simulink model "ofdm_txrx_alamouti"  (Simulink 6.3)
settings: Solver=ode45  StartTime=0.0  StopTime=45000  RelTol=1e-3
InitFcn:
  | ofdm_rx_alamouti_init
StartFcn:
  | ofdm_rx_alamouti_init
values from neighbouring files (symbols this model uses):
  CPLength = 16    [ofdm_tx_alamouti_init.m]
  CRCPolynomial = hex2dec('04c11db7')    [calcTxCRC.m]    (also in ofdm_tx_alamouti_init.m)
  hi = zeros(1,length(cci))    [ofdm_rx_alamouti_init.m]
  hr = zeros(1,length(ccr))    [ofdm_rx_alamouti_init.m]
  max_CP_length = 16    [ofdm_tx_alamouti_init.m]
  max_num_baseRateSymbols = 31    [ofdm_tx_alamouti_init.m]
  max_num_subcarriers = 64    [ofdm_tx_alamouti_init.m]
  max_num_trainingSymbols = 15    [ofdm_tx_alamouti_init.m]
  max_OFDM_symbols = 2047    [ofdm_tx_alamouti_init.m]
  modConstellation_qam16 = 0.75*[1 3 -1 -3]./3    [ofdm_tx_alamouti_init.m]
  modConstellation_qam256 = 0.9375*[3 1 5 7 11 9 13 15 -3 -1 -5 -7 -11 -9 -13 -15]./15    [ofdm_tx_alamouti_init.m]
  modConstellation_qam64 = 0.875*[3 1 5 7 -3 -1 -5 -7]./7    [ofdm_tx_alamouti_init.m]
  modConstellation_qpsk = [1 -1]./sqrt(2)    [ofdm_tx_alamouti_init.m]
  numBaseRateSymbols = 2    [ofdm_tx_alamouti_init.m]
  numFullRateSymbols = numFullRateSymbols + mod(numFullRateSymbols, 2)    [ofdm_tx_alamouti_init.m]
  numSubcarriers = 64    [ofdm_tx_alamouti_init.m]
  numTrainingSymbols = 4    [ofdm_tx_alamouti_init.m]
  pkt_crossCorr_thresh = 0.7    [ofdm_rx_alamouti_init.m]
  pkt_energy_thresh = 0    [ofdm_rx_alamouti_init.m]
  pkt_numPayloadBytes = 24+4+20+20+500    (= 568)    [ofdm_tx_alamouti_init.m]
  pktTiming_count_payloadStart = pktTiming_count_lsStart + pktTiming_ls_num*pktTiming_ls_length    [ofdm_rx_init_packetTimingControl.m]
  preamble = 6*[0 shortsyms_10 longsyms_2]    [ofdm_tx_alamouti_init.m]
  preamble_I = real(preamble)    [ofdm_tx_alamouti_init.m]
  preamble_Q = imag(preamble)    [ofdm_tx_alamouti_init.m]
  RAM_init_size = 4096    [ofdm_tx_alamouti_init.m]
  rx_longCorrCountLoad = 251-16    (= 235)    [ofdm_rx_alamouti_init.m]
  rx_longCorrThresh = 3000    [ofdm_rx_alamouti_init.m]
  RxDataScrambling_Seq = [40 198 78 63 82 173 102 245 48 111 172 115 147 230 216 93 72 65 62 2 205 242 122 90 128 83 105 97 73 10 5 252]    [ofdm_tx_alamouti_init.m]
  simOnly_numSamples = length(preamble)+( (numSubcarriers+CPLength)*(numTrainingSymbols + numBaseRateSymbols + numFullRateSymbols) )    [ofdm_tx_alamouti_init.m]
  train = [train train]    [ofdm_tx_alamouti_init.m]
  TxDataScrambling_Seq = [40 198 78 63 82 173 102 245 48 111 172 115 147 230 216 93 72 65 62 2 205 242 122 90 128 83 105 97 73 10 5 252]    [ofdm_tx_alamouti_init.m]
undefined symbols (not defined in the file or in its folder): CRC_table_gen, gcbh, interpFilter_4, xlBlockLoadCallback
libraries: xbsIndex_r4 x4373, dspmlti4 x4, dspsigops x4, commchan3 x2, simulink x2, commrflib2 x2, commsink2 x2, dspsrcs4 x1
(part 12 of 39)

== system ofdm_txrx_alamouti/OFDM Rx Alamouti/Equalizer & Packetizer/Alamouti Combining/R0*[H0*,H1*] ==
  Re[y] : Inport
  Im[y] : Inport  Port=2
  Re[h1] : Inport  Port=3
  Im[h1] : Inport  Port=4
  Re[h2] : Inport  Port=5
  Im[h2] : Inport  Port=6
  1/2 : Inport  Port=7
  ComplexMult_x1 : Subsystem
  First EQ Block : Scope  NumInputPorts=6
      settings: TimeRange=5000  YMin=0~-0.001~-0.4~-0.2~0~0  YMax=0.8~0.001~0.4~0.8~1~80  SaveName=ScopeData58
  Mux1 : xbsIndex_r4/Mux  inputs=2  en=off  latency=0  precision=Full  arith_type="Signed  (2's comp)"  n_bits=16  bin_pt=13  quantization=Truncate  overflow=Wrap  dbl_ovrd=off  block_type=mux
      settings: xl_area=[14 0 0 28 0 0 0]  block_version=VER_STRING_GOES_HERE
  Mux5 : xbsIndex_r4/Mux  inputs=2  en=off  latency=0  precision=Full  arith_type="Signed  (2's comp)"  n_bits=16  bin_pt=13  quantization=Truncate  overflow=Wrap  dbl_ovrd=off  block_type=mux
      settings: xl_area=[14 0 0 28 0 0 0]  block_version=VER_STRING_GOES_HERE
  Negate1 : xbsIndex_r4/Negate  precision=User Defined  arith_type="Signed  (2's comp)"  n_bits=18  bin_pt=16  quantization=Truncate  overflow=Flag as error  en=off  latency=0  dbl_ovrd=off  block_type=negate
      settings: xl_area=[7 0 0 14 0 0 0]  block_version=VER_STRING_GOES_HERE
  Negate2 : xbsIndex_r4/Negate  precision=User Defined  arith_type="Signed  (2's comp)"  n_bits=18  bin_pt=16  quantization=Truncate  overflow=Flag as error  en=off  latency=0  dbl_ovrd=off  block_type=negate
      settings: xl_area=[7 0 0 14 0 0 0]  block_version=VER_STRING_GOES_HERE
  done{18..22,24} (6 alike) : xbsIndex_r4/Gateway Out  hdl_port=off  timing_constraint=None  locs_specified=off  LOCs={}  block_type=gatewayout
      settings: infoedit="Gateway out block.  Converts Xilinx fixed point inputs into ouputs of type Simulink integer, double, or fixed point.<P><P>Hardware notes:  In hardware these blocks become top level output ports or are discarded, depending on how they are configured."  xl_area=[0 0 0 0 1 0 0]  block_version=VER_STRING_GOES_HERE
  Re : Outport
  Im : Outport  Port=2
  tag scope: ChanEst_I_EQ=global
  wires:
  Mux5/1 -> ComplexMult_x1/3(Re[B]), done19/1, [ChanEst_I_EQ]
  Mux1/1 -> ComplexMult_x1/4(Im[B]), done22/1
  Re[y]/1 -> ComplexMult_x1/1(Re[A]), done20/1
  ComplexMult_x1/1(Re) -> Re/1, done18/1
  Im[y]/1 -> ComplexMult_x1/2(Im[A]), done21/1
  Re[h1]/1 -> Mux5/2
  ComplexMult_x1/2(Im) -> Im/1, done24/1
  Re[h2]/1 -> Mux5/3
  Im[h1]/1 -> Negate2/1
  Im[h2]/1 -> Negate1/1
  1/2/1 -> Mux5/1, Mux1/1
  Negate1/1 -> Mux1/3
  Negate2/1 -> Mux1/2
  done18/1 -> First EQ Block/5
  done20/1 -> First EQ Block/1
  done21/1 -> First EQ Block/2
  done19/1 -> First EQ Block/3
  done22/1 -> First EQ Block/4
  done24/1 -> First EQ Block/6

== system ofdm_txrx_alamouti/OFDM Rx Alamouti/Equalizer & Packetizer/Alamouti Combining/R1* *[H1,-H0] ==
  Re[y] : Inport
  Im[y] : Inport  Port=2
  Re[h1] : Inport  Port=3
  Im[h1] : Inport  Port=4
  Re[h2] : Inport  Port=5
  Im[h2] : Inport  Port=6
  1/2 : Inport  Port=7
  ComplexMult_x1 : Subsystem
  Mux1 : xbsIndex_r4/Mux  inputs=2  en=off  latency=0  precision=Full  arith_type="Signed  (2's comp)"  n_bits=16  bin_pt=13  quantization=Truncate  overflow=Wrap  dbl_ovrd=off  block_type=mux
      settings: xl_area=[14 0 0 28 0 0 0]  block_version=VER_STRING_GOES_HERE
  Mux5 : xbsIndex_r4/Mux  inputs=2  en=off  latency=0  precision=Full  arith_type="Signed  (2's comp)"  n_bits=16  bin_pt=13  quantization=Truncate  overflow=Wrap  dbl_ovrd=off  block_type=mux
      settings: xl_area=[14 0 0 28 0 0 0]  block_version=VER_STRING_GOES_HERE
  Negate1 : xbsIndex_r4/Negate  precision=User Defined  arith_type="Signed  (2's comp)"  n_bits=18  bin_pt=16  quantization=Truncate  overflow=Flag as error  en=off  latency=0  dbl_ovrd=off  block_type=negate
      settings: xl_area=[7 0 0 14 0 0 0]  block_version=VER_STRING_GOES_HERE
  Negate2 : xbsIndex_r4/Negate  precision=User Defined  arith_type="Signed  (2's comp)"  n_bits=18  bin_pt=16  quantization=Truncate  overflow=Flag as error  en=off  latency=0  dbl_ovrd=off  block_type=negate
      settings: xl_area=[7 0 0 14 0 0 0]  block_version=VER_STRING_GOES_HERE
  Negate3 : xbsIndex_r4/Negate  precision=User Defined  arith_type="Signed  (2's comp)"  n_bits=16  bin_pt=15  quantization=Truncate  overflow=Flag as error  en=off  latency=0  dbl_ovrd=off  block_type=negate
      settings: xl_area=[7 0 0 14 0 0 0]  block_version=VER_STRING_GOES_HERE
  Second EQ Block : Scope  NumInputPorts=6
      settings: TimeRange=5000  YMin=0~-0.001~-0.4~-0.2~0~0  YMax=0.8~0.001~0.4~0.8~1~80  SaveName=ScopeData62
  done{18..22,24} (6 alike) : xbsIndex_r4/Gateway Out  hdl_port=off  timing_constraint=None  locs_specified=off  LOCs={}  block_type=gatewayout
      settings: infoedit="Gateway out block.  Converts Xilinx fixed point inputs into ouputs of type Simulink integer, double, or fixed point.<P><P>Hardware notes:  In hardware these blocks become top level output ports or are discarded, depending on how they are configured."  xl_area=[0 0 0 0 1 0 0]  block_version=VER_STRING_GOES_HERE
  Re : Outport
  Im : Outport  Port=2
  wires:
  1/2/1 -> Mux1/1, Mux5/1
  Im[h2]/1 -> Negate2/1
  Im[h1]/1 -> Mux1/2
  Re[h2]/1 -> Negate1/1
  ComplexMult_x1/2(Im) -> Im/1, done24/1
  Re[h1]/1 -> Mux5/2
  Im[y]/1 -> Negate3/1
  ComplexMult_x1/1(Re) -> Re/1, done18/1
  Re[y]/1 -> ComplexMult_x1/1(Re[A]), done20/1
  Mux1/1 -> ComplexMult_x1/4(Im[B]), done22/1
  Mux5/1 -> ComplexMult_x1/3(Re[B]), done19/1
  Negate1/1 -> Mux5/3
  Negate2/1 -> Mux1/3
  Negate3/1 -> ComplexMult_x1/2(Im[A]), done21/1
  done18/1 -> Second EQ Block/5
  done20/1 -> Second EQ Block/1
  done21/1 -> Second EQ Block/2
  done19/1 -> Second EQ Block/3
  done22/1 -> Second EQ Block/4
  done24/1 -> Second EQ Block/6

== system ofdm_txrx_alamouti/OFDM Rx Alamouti/Equalizer & Packetizer/Packet_Constructor/   ==
  signal : Inport
  Delay1 : xbsIndex_r4/Delay  en=off  latency=1  dbl_ovrd=off  reg_retiming=off  block_type=delay
      settings: infoedit=Hardware notes: A delay line is a chain, each link of which is an SRL16 followed by a flip-flop. If register retiming is enabled, the delay line is a chain of flip-flops.  xl_area=[1 1 0 0 0 0 0]  block_version=VER_STRING_GOES_HERE
  Inverter1 : xbsIndex_r4/Inverter  en=off  latency=0  dbl_ovrd=off  block_type=inv
      settings: xl_area=[0 0 0 0 0 0 0]  block_version=VER_STRING_GOES_HERE
  Logical1 : xbsIndex_r4/Logical  logical_function=AND  inputs=2  en=off  latency=0  precision=Full  arith_type=Unsigned  n_bits=8  bin_pt=2  align_bp=on  dbl_ovrd=off  block_type=logical
      settings: xl_area=[1 0 0 1 0 0 0]  block_version=VER_STRING_GOES_HERE
  pulse : Outport
  wires:
  Inverter1/1 -> Delay1/1
  signal/1 -> Inverter1/1, Logical1/1
  Delay1/1 -> Logical1/2
  Logical1/1 -> pulse/1

== system ofdm_txrx_alamouti/OFDM Rx Alamouti/Equalizer & Packetizer/Packet_Constructor/Barrel Shifter ==
  ShiftSel : Inport
  D : Inport  Port=2
  en : Inport  Port=3
  AddSub1 : xbsIndex_r4/AddSub  mode=Addition  use_carryin=off  use_carryout=off  en=off  latency=0  precision=User Defined  arith_type=Unsigned  n_bits=15  bin_pt=0  quantization=Truncate  overflow=Wrap  dbl_ovrd=off  use_behavioral_HDL=on  pipelined=off  use_rpm=on  block_type=addsub
      settings: xl_area=[8 0 0 15 0 0 0]  block_version=VER_STRING_GOES_HERE
  Assert2 : xbsIndex_r4/Assert  Orientation=down  assert_type=off  type_source=Explicitly  arith_type="Signed  (2's comp)"  n_bits=16  bin_pt=14  assert_rate=on  rate_source=Explicitly  period=2  output_port=on  block_type=assert
      settings: infoedit=Asserts a user-defined sample rate and/or type on a signal.<P><P>Hardware notes: In hardware this block costs nothing.  block_version=VER_STRING_GOES_HERE
  Data Buffer : xbsIndex_r4/Register  Orientation=left  init=0  rst=off  en=on  dbl_ovrd=off  block_type=register
      settings: xl_area=[8 15 0 0 0 0 0]  block_version=VER_STRING_GOES_HERE
  Delay2 : xbsIndex_r4/Delay  en=off  latency=1  dbl_ovrd=off  reg_retiming=off  block_type=delay
      settings: infoedit=Hardware notes: A delay line is a chain, each link of which is an SRL16 followed by a flip-flop. If register retiming is enabled, the delay line is a chain of flip-flops.  xl_area=[1 1 0 0 0 0 0]  block_version=VER_STRING_GOES_HERE
  Shift : xbsIndex_r4/Shift  shift_dir=Left  shift_bits=1  en=off  latency=0  precision=User Defined  arith_type=Unsigned  n_bits=15  bin_pt=0  quantization=Truncate  overflow=Wrap  dbl_ovrd=off  block_type=shift
      settings: xl_area=[0 0 0 0 0 0 0]  block_version=VER_STRING_GOES_HERE
  Shift1 : xbsIndex_r4/Shift  shift_dir=Left  shift_bits=2  en=off  latency=0  precision=User Defined  arith_type=Unsigned  n_bits=15  bin_pt=0  quantization=Truncate  overflow=Wrap  dbl_ovrd=off  block_type=shift
      settings: xl_area=[0 0 0 0 0 0 0]  block_version=VER_STRING_GOES_HERE
  Shift2 : xbsIndex_r4/Shift  shift_dir=Left  shift_bits=4  en=off  latency=0  precision=User Defined  arith_type=Unsigned  n_bits=15  bin_pt=0  quantization=Truncate  overflow=Wrap  dbl_ovrd=off  block_type=shift
      settings: xl_area=[0 0 0 0 0 0 0]  block_version=VER_STRING_GOES_HERE
  Shift3 : xbsIndex_r4/Shift  shift_dir=Left  shift_bits=6  en=off  latency=0  precision=User Defined  arith_type=Unsigned  n_bits=15  bin_pt=0  quantization=Truncate  overflow=Wrap  dbl_ovrd=off  block_type=shift
      settings: xl_area=[0 0 0 0 0 0 0]  block_version=VER_STRING_GOES_HERE
  Shift4 : xbsIndex_r4/Shift  shift_dir=Left  shift_bits=8  en=off  latency=0  precision=User Defined  arith_type=Unsigned  n_bits=15  bin_pt=0  quantization=Truncate  overflow=Wrap  dbl_ovrd=off  block_type=shift
      settings: xl_area=[0 0 0 0 0 0 0]  block_version=VER_STRING_GOES_HERE
  Shift5 : xbsIndex_r4/Shift  shift_dir=Left  shift_bits=3  en=off  latency=0  precision=User Defined  arith_type=Unsigned  n_bits=15  bin_pt=0  quantization=Truncate  overflow=Wrap  dbl_ovrd=off  block_type=shift
      settings: xl_area=[0 0 0 0 0 0 0]  block_version=VER_STRING_GOES_HERE
  Shift6 : xbsIndex_r4/Shift  shift_dir=Left  shift_bits=5  en=off  latency=0  precision=User Defined  arith_type=Unsigned  n_bits=15  bin_pt=0  quantization=Truncate  overflow=Wrap  dbl_ovrd=off  block_type=shift
      settings: xl_area=[0 0 0 0 0 0 0]  block_version=VER_STRING_GOES_HERE
  Shift7 : xbsIndex_r4/Shift  shift_dir=Left  shift_bits=7  en=off  latency=0  precision=User Defined  arith_type=Unsigned  n_bits=15  bin_pt=0  quantization=Truncate  overflow=Wrap  dbl_ovrd=off  block_type=shift
      settings: xl_area=[0 0 0 0 0 0 0]  block_version=VER_STRING_GOES_HERE
  shift selector : xbsIndex_r4/Mux  inputs=9  en=off  latency=0  precision=Full  arith_type="Signed  (2's comp)"  n_bits=8  bin_pt=2  quantization=Truncate  overflow=Wrap  dbl_ovrd=off  block_type=mux
      settings: xl_area=[68 0 0 135 0 0 0]  block_version=VER_STRING_GOES_HERE
  Q : Outport
  wires:
  D/1 -> Delay2/1
  ShiftSel/1 -> shift selector/1
  Shift7/1 -> shift selector/9
  Shift6/1 -> shift selector/7
  Shift5/1 -> shift selector/5
  shift selector/1 -> AddSub1/2
  Shift4/1 -> shift selector/10
  Shift3/1 -> shift selector/8
  Shift2/1 -> shift selector/6
  Shift1/1 -> shift selector/4
  Shift/1 -> shift selector/3
  AddSub1/1 -> Assert2/1
  Data Buffer/1 -> Shift5/1, shift selector/2, Shift/1, Shift1/1, Shift2/1, Shift6/1, Shift3/1, Shift7/1, Shift4/1
  en/1 -> Data Buffer/2
  Delay2/1 -> AddSub1/1
  Assert2/1 -> Q/1, Data Buffer/1

== system ofdm_txrx_alamouti/OFDM Rx Alamouti/Equalizer & Packetizer/Packet_Constructor/Data Buffer ==
  Payload_Addr : Inport
  Payload_WE : Inport  Port=2
  Payload_Data : Inport  Port=3
  Rst : Inport  Port=4
  BER Calc : Subsystem
  CRC Calc : Subsystem
  Descrambling & Pkt Buffer : Subsystem
  Header Decoding : Subsystem
  Rx Bytes : Scope  NumInputPorts=5
      settings: TimeRange=1800  YMin=0~0~540~1330~-1  YMax=1~275~552.5~1470~1  SaveName=ScopeData33
  done{1..2,6..7,9} (5 alike) : xbsIndex_r4/Gateway Out  hdl_port=off  timing_constraint=None  locs_specified=off  LOCs={}  block_type=gatewayout
      settings: infoedit="Gateway out block.  Converts Xilinx fixed point inputs into ouputs of type Simulink integer, double, or fixed point.<P><P>Hardware notes:  In hardware these blocks become top level output ports or are discarded, depending on how they are configured."  xl_area=[0 0 0 0 1 0 0]  block_version=VER_STRING_GOES_HERE
  numBytes : Outport
  modMask : Outport  Port=2
  CRCError : Outport  Port=3
  HdrError : Outport  Port=4
  tag scope: BER_Errors=global, BER_TotalBits=global
  wires:
  done6/1 -> Rx Bytes/1
  done7/1 -> Rx Bytes/2
  done1/1 -> Rx Bytes/3
  done9/1 -> Rx Bytes/5
  Payload_Addr/1 -> Descrambling & Pkt Buffer/1(Addr)
  Payload_Data/1 -> Descrambling & Pkt Buffer/2(Data)
  Payload_WE/1 -> Descrambling & Pkt Buffer/3(We)
  Rst/1 -> Header Decoding/4(Rst), CRC Calc/5(Rst)
  CRC Calc/1(PktError) -> CRCError/1, done9/1
  BER Calc/2(BER_tot_32LSB) -> [BER_TotalBits]
  BER Calc/1(BER_errs) -> [BER_Errors]
  Descrambling & Pkt Buffer/1(" Addr") -> BER Calc/1(BER_addr), Header Decoding/1(RxByteAddr), CRC Calc/3(ByteAddr), done1/1
  Descrambling & Pkt Buffer/2(" Data") -> BER Calc/2(BER_data), done7/1, CRC Calc/1(ByteIn), Header Decoding/2(RxByte)
  Descrambling & Pkt Buffer/3(" Vout") -> BER Calc/3(BER_val), CRC Calc/2(Vin), Header Decoding/3(RxByteWE), done6/1
  Header Decoding/1(numBytes) -> numBytes/1, done2/1, BER Calc/4(numBytes), CRC Calc/4(NumBytes)
  done2/1 -> Rx Bytes/4
  Header Decoding/2(modMask) -> modMask/1
  CRC Calc/2(HdrError) -> HdrError/1

== system ofdm_txrx_alamouti/OFDM Rx Alamouti/Equalizer & Packetizer/Packet_Constructor/Detection Disable ==
  CRC-Err : Inport
  PktDone : Inport  Port=2
  HdrError : Inport  Port=3
  Concat : xbsIndex_r4/Concat  num_inputs=2  dbl_ovrd=off  block_type=concat
      settings: block_version=VER_STRING_GOES_HERE
  Constant : xbsIndex_r4/Constant  arith_type=Boolean  const=1  n_bits=16  bin_pt=14  explicit_period=off  period=1  equ=P=C  opselect=C  inp2=PCIN>>17  opr=+  inp1=P  carry=CIN  dbl_ovrd=off  block_type=constant
      settings: block_version=VER_STRING_GOES_HERE
  Good-Bad Pkt Intterupts : Scope  NumInputPorts=6
      settings: YMin=-1~-5~-5~-5~-5~-5  YMax=1~5~5~5~5~5  SaveName=ScopeData68
  Inverter : xbsIndex_r4/Inverter  en=off  latency=0  dbl_ovrd=off  block_type=inv
      settings: xl_area=[0 0 0 0 0 0 0]  block_version=VER_STRING_GOES_HERE
  LSB+0 : xbsIndex_r4/Slice  nbits=1  boolean_output=on  mode=Lower Bit Location + Width  bit1=0  base1=MSB of Input  bit0=0  base0=LSB of Input  dbl_ovrd=off  block_type=slice
      settings: infoedit="Extracts a given range of bits from each input sample and presents it at the output.  The output type is ordinarily unsigned with binary point at zero, but can be Boolean when the slice is one bit wide.<P><P>Hardware notes: In hardware this block costs nothing."  block_version=VER_STRING_GOES_HERE
  LSB+1 : xbsIndex_r4/Slice  nbits=1  boolean_output=on  mode=Lower Bit Location + Width  bit1=0  base1=MSB of Input  bit0=1  base0=LSB of Input  dbl_ovrd=off  block_type=slice
      settings: infoedit="Extracts a given range of bits from each input sample and presents it at the output.  The output type is ordinarily unsigned with binary point at zero, but can be Boolean when the slice is one bit wide.<P><P>Hardware notes: In hardware this block costs nothing."  block_version=VER_STRING_GOES_HERE
  LSB+4 : xbsIndex_r4/Slice  nbits=1  boolean_output=off  mode=Lower Bit Location + Width  bit1=0  base1=MSB of Input  bit0=4  base0=LSB of Input  dbl_ovrd=off  block_type=slice
      settings: infoedit="Extracts a given range of bits from each input sample and presents it at the output.  The output type is ordinarily unsigned with binary point at zero, but can be Boolean when the slice is one bit wide.<P><P>Hardware notes: In hardware this block costs nothing."  block_version=VER_STRING_GOES_HERE
  LSB+5 : xbsIndex_r4/Slice  nbits=1  boolean_output=off  mode=Lower Bit Location + Width  bit1=0  base1=MSB of Input  bit0=5  base0=LSB of Input  dbl_ovrd=off  block_type=slice
      settings: infoedit="Extracts a given range of bits from each input sample and presents it at the output.  The output type is ordinarily unsigned with binary point at zero, but can be Boolean when the slice is one bit wide.<P><P>Hardware notes: In hardware this block costs nothing."  block_version=VER_STRING_GOES_HERE
  Logical{1..2,5..6} (4 alike) : xbsIndex_r4/Logical  logical_function=OR  inputs=2  en=off  latency=0  precision=Full  arith_type=Unsigned  n_bits=16  bin_pt=0  align_bp=on  dbl_ovrd=off  block_type=logical
      settings: block_version=VER_STRING_GOES_HERE
  Logical3 : xbsIndex_r4/Logical  logical_function=AND  inputs=2  en=off  latency=0  precision=Full  arith_type=Unsigned  n_bits=16  bin_pt=0  align_bp=on  dbl_ovrd=off  block_type=logical
      settings: block_version=VER_STRING_GOES_HERE
  Logical4 : xbsIndex_r4/Logical  logical_function=AND  inputs=2  en=off  latency=0  precision=Full  arith_type=Unsigned  n_bits=16  bin_pt=0  align_bp=on  dbl_ovrd=off  block_type=logical
      settings: block_version=VER_STRING_GOES_HERE
  Posedge : Subsystem
  Posedge1 : Subsystem
  Register1 : xbsIndex_r4/Register  init=0  rst=on  en=on  dbl_ovrd=off  block_type=register
      settings: xl_area=[1 1 0 0 0 0 0]  block_version=VER_STRING_GOES_HERE
  Register2 : xbsIndex_r4/Register  init=0  rst=on  en=on  dbl_ovrd=off  block_type=register
      settings: xl_area=[1 1 0 0 0 0 0]  block_version=VER_STRING_GOES_HERE
  Rx _Int_goodPkt : xbsIndex_r4/Gateway Out  hdl_port=on  timing_constraint=None  locs_specified=off  LOCs={}  block_type=gatewayout
      settings: infoedit="Gateway out block.  Converts Xilinx fixed point inputs into ouputs of type Simulink integer, double, or fixed point.<P><P>Hardware notes:  In hardware these blocks become top level output ports or are discarded, depending on how they are configured."  xl_area=[0 0 0 0 1 0 0]  block_version=VER_STRING_GOES_HERE
  Rx _Int_goodPkt{1..5} (5 alike) : xbsIndex_r4/Gateway Out  hdl_port=off  timing_constraint=None  locs_specified=off  LOCs={}  block_type=gatewayout
      settings: infoedit="Gateway out block.  Converts Xilinx fixed point inputs into ouputs of type Simulink integer, double, or fixed point.<P><P>Hardware notes:  In hardware these blocks become top level output ports or are discarded, depending on how they are configured."  xl_area=[0 0 0 0 1 0 0]  block_version=VER_STRING_GOES_HERE
  Rx_Int_badPkt : xbsIndex_r4/Gateway Out  hdl_port=on  timing_constraint=None  locs_specified=off  LOCs={}  block_type=gatewayout
      settings: infoedit="Gateway out block.  Converts Xilinx fixed point inputs into ouputs of type Simulink integer, double, or fixed point.<P><P>Hardware notes:  In hardware these blocks become top level output ports or are discarded, depending on how they are configured."  xl_area=[0 0 0 0 1 0 0]  block_version=VER_STRING_GOES_HERE
  Rx_packet_done : xbsIndex_r4/To Register  shared_memory_name='Rx_packet_done'  init=0  ownership=Locally owned and initialized  explicit_data_type=off  arith_type="Signed  (2's comp)"  n_bits=16  bin_pt=14  dbl_ovrd=off  block_type=toreg
      settings: AttributesFormatString=<< %<shared_memory_name> >>  infoedit="Register block that writes data to a shared memory register.  Delay of one sample period."  block_version=VER_STRING_GOES_HERE
  Scope : Scope
      settings: YMin=-1  YMax=1  SaveName=ScopeData51
  Up Sample : xbsIndex_r4/Up Sample  sample_ratio=2  copy_samples=on  dbl_ovrd=off  block_type=usamp
      settings: infoedit="Up samples input data.  Inserted values  can be zeros or copies of the most recent input sample.<P><P>Hardware notes: No hardware is needed if inserted values are copies of the input sample; otherwise, a mux and single bit flip-flop are used."  block_version=VER_STRING_GOES_HERE
  Up Sample1 : xbsIndex_r4/Up Sample  sample_ratio=2  copy_samples=on  dbl_ovrd=off  block_type=usamp
      settings: infoedit="Up samples input data.  Inserted values  can be zeros or copies of the most recent input sample.<P><P>Hardware notes: No hardware is needed if inserted values are copies of the input sample; otherwise, a mux and single bit flip-flop are used."  block_version=VER_STRING_GOES_HERE
  Up Sample2 : xbsIndex_r4/Up Sample  sample_ratio=2  copy_samples=on  dbl_ovrd=off  block_type=usamp
      settings: infoedit="Up samples input data.  Inserted values  can be zeros or copies of the most recent input sample.<P><P>Hardware notes: No hardware is needed if inserted values are copies of the input sample; otherwise, a mux and single bit flip-flop are used."  block_version=VER_STRING_GOES_HERE
  rst_out : Outport
  tag scope: BadPktInt=global, GoodPktInt=global, Rx_GoodPktInterrupt=global
  wires:
  LSB+0/1 -> Posedge/1(D)
  LSB+1/1 -> Posedge1/1(D)
  LSB+4/1 -> Register2/1
  LSB+5/1 -> Register1/1
  PktDone/1 -> Logical3/1, Logical4/1, Rx _Int_goodPkt2/1
  [regRx_PktDone_Reset] -> LSB+0/1, LSB+1/1, LSB+4/1, LSB+5/1
  Logical6/1 -> Inverter/1, Logical4/2, Rx _Int_goodPkt3/1
  Inverter/1 -> Logical3/2
  Logical5/1 -> Register2/2
  Logical2/1 -> Register1/2
  Posedge/1(Q) -> Logical5/1, Logical1/1
  Posedge1/1(Q) -> Logical2/2, Logical1/2
  [rx_global_reset_DV2] -> Logical5/2, Logical2/1
  Logical3/1 -> Register2/3, Rx _Int_goodPkt4/1
  Logical4/1 -> Register1/3, Rx _Int_goodPkt5/1
  Register2/1 -> Concat/2, Up Sample1/1, [GoodPktInt], [Rx_GoodPktInterrupt]
  Register1/1 -> Concat/1, [BadPktInt], Up Sample2/1
  Constant/1 -> Rx_packet_done/2
  Concat/1 -> Up Sample/1
  Logical1/1 -> rst_out/1
  Up Sample/1 -> Rx_packet_done/1, Rx _Int_goodPkt1/1
  Rx _Int_goodPkt1/1 -> Scope/1
  Rx _Int_goodPkt2/1 -> Good-Bad Pkt Intterupts/1
  Rx _Int_goodPkt3/1 -> Good-Bad Pkt Intterupts/2
  Rx _Int_goodPkt4/1 -> Good-Bad Pkt Intterupts/3
  Rx _Int_goodPkt5/1 -> Good-Bad Pkt Intterupts/4
  Rx _Int_goodPkt/1 -> Good-Bad Pkt Intterupts/5
  Rx_Int_badPkt/1 -> Good-Bad Pkt Intterupts/6
  Up Sample1/1 -> Rx _Int_goodPkt/1
  Up Sample2/1 -> Rx_Int_badPkt/1
  CRC-Err/1 -> Logical6/2
  HdrError/1 -> Logical6/1

== system ofdm_txrx_alamouti/OFDM Rx Alamouti/Equalizer & Packetizer/Packet_Constructor/Hard Decision ==
  note: This block demaps the signal according to its modulation scheme by slicing off a certain number of most signicant bits and then using that sliced number for a lookup table that
      | reverses the grey coding and produces the bit values.
  note: [0 1 3 2]
  Mod Sel : Inport
  I : Inport  Port=2
  Q : Inport  Port=3
  Concat{,1,5..6} (4 alike) : xbsIndex_r4/Concat  num_inputs=2  dbl_ovrd=off  block_type=concat
      settings: block_version=VER_STRING_GOES_HERE
  Constant7 : xbsIndex_r4/Constant  arith_type=Unsigned  const=0  n_bits=8  bin_pt=0  explicit_period=off  period=1  equ=P=C  opselect=C  inp2=PCIN>>17  opr=+  inp1=P  carry=CIN  dbl_ovrd=off  block_type=constant
      settings: block_version=VER_STRING_GOES_HERE
  Delay{,1,4..5} (4 alike) : xbsIndex_r4/Delay  en=off  latency=1  dbl_ovrd=off  reg_retiming=off  block_type=delay
      settings: infoedit=Hardware notes: A delay line is a chain, each link of which is an SRL16 followed by a flip-flop. If register retiming is enabled, the delay line is a chain of flip-flops.  xl_area=[2 4 0 0 0 0 0]  block_version=VER_STRING_GOES_HERE
  Mux2 : xbsIndex_r4/Mux  inputs=9  en=off  latency=0  precision=User Defined  arith_type=Unsigned  n_bits=8  bin_pt=0  quantization=Truncate  overflow=Wrap  dbl_ovrd=off  block_type=mux
      settings: xl_area=[36 0 0 72 0 0 0]  block_version=VER_STRING_GOES_HERE
  Pkt Constructor Hard Decision : Scope  NumInputPorts=5
      settings: TimeRange=8000  YMin=0~-1~-0.4~0~0  YMax=4~1~0.4~3~10  SaveName=ScopeData35
  ROM : xbsIndex_r4/ROM  depth=4  initVector=[0 1 3 2]  distributed_mem=Distributed memory  rst=off  init_reg=0  en=off  latency=1  arith_type=Unsigned  n_bits=2  bin_pt=0  dbl_ovrd=off  optimize=Area  use_rpm=off  block_type=sprom
      settings: xl_area=[1 2 0 2 0 0 0]  block_version=VER_STRING_GOES_HERE
  ROM1 : xbsIndex_r4/ROM  depth=4  initVector=[0 1 3 2]  distributed_mem=Distributed memory  rst=off  init_reg=0  en=off  latency=1  arith_type=Unsigned  n_bits=2  bin_pt=0  dbl_ovrd=off  optimize=Area  use_rpm=off  block_type=sprom
      settings: xl_area=[1 2 0 2 0 0 0]  block_version=VER_STRING_GOES_HERE
  ROM2 : xbsIndex_r4/ROM  depth=8  initVector=[1 0 2 3 7 6 4 5]  distributed_mem=Distributed memory  rst=off  init_reg=0  en=off  latency=1  arith_type=Unsigned  n_bits=3  bin_pt=0  dbl_ovrd=off  optimize=Area  use_rpm=off  block_type=sprom
      settings: xl_area=[2 3 0 3 0 0 0]  block_version=VER_STRING_GOES_HERE
  ROM3 : xbsIndex_r4/ROM  depth=8  initVector=[1 0 2 3 7 6 4 5]  distributed_mem=Distributed memory  rst=off  init_reg=0  en=off  latency=1  arith_type=Unsigned  n_bits=3  bin_pt=0  dbl_ovrd=off  optimize=Area  use_rpm=off  block_type=sprom
      settings: xl_area=[2 3 0 3 0 0 0]  block_version=VER_STRING_GOES_HERE
  ROM4 : xbsIndex_r4/ROM  depth=16  initVector=[1 0 2 3 5 4 6 7 15 14 12 13 11 10 8 9]  distributed_mem=Distributed memory  rst=off  init_reg=0  en=off  latency=1  arith_type=Unsigned  n_bits=4  bin_pt=0  dbl_ovrd=off  optimize=Area  use_rpm=off  block_type=sprom
      settings: xl_area=[2 4 0 4 0 0 0]  block_version=VER_STRING_GOES_HERE
  ROM5 : xbsIndex_r4/ROM  depth=16  initVector=[1 0 2 3 5 4 6 7 15 14 12 13 11 10 8 9]  distributed_mem=Distributed memory  rst=off  init_reg=0  en=off  latency=1  arith_type=Unsigned  n_bits=4  bin_pt=0  dbl_ovrd=off  optimize=Area  use_rpm=off  block_type=sprom
      settings: xl_area=[2 4 0 4 0 0 0]  block_version=VER_STRING_GOES_HERE
  Slice : xbsIndex_r4/Slice  nbits=1  boolean_output=off  mode=Upper Bit Location + Width  bit1=0  base1=MSB of Input  bit0=0  base0=LSB of Input  dbl_ovrd=off  block_type=slice
      settings: infoedit="Extracts a given range of bits from each input sample and presents it at the output.  The output type is ordinarily unsigned with binary point at zero, but can be Boolean when the slice is one bit wide.<P><P>Hardware notes: In hardware this block costs nothing."  block_version=VER_STRING_GOES_HERE
  Slice1 : xbsIndex_r4/Slice  nbits=1  boolean_output=off  mode=Upper Bit Location + Width  bit1=0  base1=MSB of Input  bit0=0  base0=LSB of Input  dbl_ovrd=off  block_type=slice
      settings: infoedit="Extracts a given range of bits from each input sample and presents it at the output.  The output type is ordinarily unsigned with binary point at zero, but can be Boolean when the slice is one bit wide.<P><P>Hardware notes: In hardware this block costs nothing."  block_version=VER_STRING_GOES_HERE
  Slice2 : xbsIndex_r4/Slice  nbits=2  boolean_output=off  mode=Upper Bit Location + Width  bit1=0  base1=MSB of Input  bit0=0  base0=LSB of Input  dbl_ovrd=off  block_type=slice
      settings: infoedit="Extracts a given range of bits from each input sample and presents it at the output.  The output type is ordinarily unsigned with binary point at zero, but can be Boolean when the slice is one bit wide.<P><P>Hardware notes: In hardware this block costs nothing."  block_version=VER_STRING_GOES_HERE
  Slice3 : xbsIndex_r4/Slice  nbits=2  boolean_output=off  mode=Upper Bit Location + Width  bit1=0  base1=MSB of Input  bit0=0  base0=LSB of Input  dbl_ovrd=off  block_type=slice
      settings: infoedit="Extracts a given range of bits from each input sample and presents it at the output.  The output type is ordinarily unsigned with binary point at zero, but can be Boolean when the slice is one bit wide.<P><P>Hardware notes: In hardware this block costs nothing."  block_version=VER_STRING_GOES_HERE
  Slice4 : xbsIndex_r4/Slice  nbits=3  boolean_output=off  mode=Upper Bit Location + Width  bit1=0  base1=MSB of Input  bit0=0  base0=LSB of Input  dbl_ovrd=off  block_type=slice
      settings: infoedit="Extracts a given range of bits from each input sample and presents it at the output.  The output type is ordinarily unsigned with binary point at zero, but can be Boolean when the slice is one bit wide.<P><P>Hardware notes: In hardware this block costs nothing."  block_version=VER_STRING_GOES_HERE
  Slice5 : xbsIndex_r4/Slice  nbits=3  boolean_output=off  mode=Upper Bit Location + Width  bit1=0  base1=MSB of Input  bit0=0  base0=LSB of Input  dbl_ovrd=off  block_type=slice
      settings: infoedit="Extracts a given range of bits from each input sample and presents it at the output.  The output type is ordinarily unsigned with binary point at zero, but can be Boolean when the slice is one bit wide.<P><P>Hardware notes: In hardware this block costs nothing."  block_version=VER_STRING_GOES_HERE
  Slice{6..9} (4 alike) : xbsIndex_r4/Slice  nbits=4  boolean_output=off  mode=Upper Bit Location + Width  bit1=0  base1=MSB of Input  bit0=0  base0=LSB of Input  dbl_ovrd=off  block_type=slice
      settings: infoedit="Extracts a given range of bits from each input sample and presents it at the output.  The output type is ordinarily unsigned with binary point at zero, but can be Boolean when the slice is one bit wide.<P><P>Hardware notes: In hardware this block costs nothing."  block_version=VER_STRING_GOES_HERE
  done{1..3,6..7} (5 alike) : xbsIndex_r4/Gateway Out  hdl_port=off  timing_constraint=None  locs_specified=off  LOCs={}  block_type=gatewayout
      settings: infoedit="Gateway out block.  Converts Xilinx fixed point inputs into ouputs of type Simulink integer, double, or fixed point.<P><P>Hardware notes:  In hardware these blocks become top level output ports or are discarded, depending on how they are configured."  xl_area=[0 0 0 0 1 0 0]  block_version=VER_STRING_GOES_HERE
  bits : Outport
  wires:
  Delay/1 -> Concat/1, Mux2/3
  Delay1/1 -> Concat/2
  Slice9/1 -> Slice/1, Slice4/1, Slice6/1, Slice2/1
  Slice8/1 -> Slice5/1, Slice7/1, Slice3/1, Slice1/1
  Slice5/1 -> ROM3/1
  Slice4/1 -> ROM2/1
  ROM3/1 -> Concat6/2
  ROM2/1 -> Concat6/1
  ROM1/1 -> Concat5/2
  ROM/1 -> Concat5/1
  ROM5/1 -> Concat1/2
  ROM4/1 -> Concat1/1
  Slice7/1 -> ROM5/1
  Slice6/1 -> ROM4/1
  Concat1/1 -> Mux2/10
  Constant7/1 -> Mux2/7, Mux2/5, Mux2/2, Mux2/9
  Mux2/1 -> bits/1, done3/1
  Mod Sel/1 -> Mux2/1, done6/1
  Concat6/1 -> Mux2/8
  Concat5/1 -> Mux2/6
  Slice3/1 -> ROM1/1
  Slice2/1 -> ROM/1
  Concat/1 -> Mux2/4, done2/1
  Q/1 -> Slice8/1, Delay5/1
  Slice1/1 -> Delay1/1
  Slice/1 -> Delay/1
  I/1 -> Slice9/1, Delay4/1
  done6/1 -> Pkt Constructor Hard Decision/1
  done7/1 -> Pkt Constructor Hard Decision/2
  done1/1 -> Pkt Constructor Hard Decision/3
  done2/1 -> Pkt Constructor Hard Decision/4
  done3/1 -> Pkt Constructor Hard Decision/5
  Delay4/1 -> done7/1
  Delay5/1 -> done1/1

== system ofdm_txrx_alamouti/OFDM Rx Alamouti/Equalizer & Packetizer/Packet_Constructor/Modulation RAM ==
  Xk_index : Inport
  Sym Index : Inport  Port=2
  FullRateMask : Inport  Port=3
  4LSB : xbsIndex_r4/Slice  nbits=4  boolean_output=off  mode=Lower Bit Location + Width  bit1=0  base1=MSB of Input  bit0=0  base0=LSB of Input  dbl_ovrd=off  block_type=slice
      settings: infoedit="Extracts a given range of bits from each input sample and presents it at the output.  The output type is ordinarily unsigned with binary point at zero, but can be Boolean when the slice is one bit wide.<P><P>Hardware notes: In hardware this block costs nothing."  block_version=VER_STRING_GOES_HERE
  Addr Gen : Subsystem
  Concat : xbsIndex_r4/Concat  num_inputs=4  dbl_ovrd=off  block_type=concat
      settings: block_version=VER_STRING_GOES_HERE
  Concat1 : xbsIndex_r4/Concat  num_inputs=4  dbl_ovrd=off  block_type=concat
      settings: block_version=VER_STRING_GOES_HERE
  Constant : Constant  Value=0
  Constant4 : xbsIndex_r4/Constant  arith_type=Boolean  const=0  n_bits=1  bin_pt=0  explicit_period=off  period=1  equ=P=C  opselect=C  inp2=PCIN>>17  opr=+  inp1=P  carry=CIN  dbl_ovrd=off  block_type=constant
      settings: block_version=VER_STRING_GOES_HERE
  Constant7 : xbsIndex_r4/Constant  arith_type=Unsigned  const=0  n_bits=4  bin_pt=0  explicit_period=off  period=1  equ=P=C  opselect=C  inp2=PCIN>>17  opr=+  inp1=P  carry=CIN  dbl_ovrd=off  block_type=constant
      settings: block_version=VER_STRING_GOES_HERE
  Inverter : xbsIndex_r4/Inverter  en=off  latency=1  dbl_ovrd=off  block_type=inv
      settings: block_version=VER_STRING_GOES_HERE
  Inverter1 : xbsIndex_r4/Inverter  en=off  latency=0  dbl_ovrd=off  block_type=inv
      settings: block_version=VER_STRING_GOES_HERE
  Logical : xbsIndex_r4/Logical  logical_function=AND  inputs=3  en=off  latency=0  precision=Full  arith_type=Unsigned  n_bits=16  bin_pt=0  align_bp=on  dbl_ovrd=off  block_type=logical
      settings: block_version=VER_STRING_GOES_HERE
  Logical1 : xbsIndex_r4/Logical  logical_function=OR  inputs=2  en=off  latency=0  precision=Full  arith_type=Unsigned  n_bits=16  bin_pt=0  align_bp=on  dbl_ovrd=off  block_type=logical
      settings: block_version=VER_STRING_GOES_HERE
  Logical3 : xbsIndex_r4/Logical  logical_function=OR  inputs=2  en=off  latency=0  precision=Full  arith_type=Unsigned  n_bits=16  bin_pt=0  align_bp=on  dbl_ovrd=off  block_type=logical
      settings: block_version=VER_STRING_GOES_HERE
  SMWOAddr_RxModulation : xbsIndex_r4/Gateway In  arith_type=Unsigned  n_bits=ceil(log2(2*numSubcarriers))-3  bin_pt=0  quantization="Round  (unbiased: +/- Inf)"  overflow=Saturate  period=4  dbl_ovrd=off  timing_constraint=None  locs_specified=off  LOCs={}  block_type=gatewayin
      settings: infoedit="Gateway in block.  Converts inputs of type Simulink integer, double and fixed point to  Xilinx fixed point type.<P><P>Hardware notes:  In hardware these blocks become top level input ports."  block_version=VER_STRING_GOES_HERE
  SMWODataI_RxModulation : xbsIndex_r4/Gateway In  arith_type=Unsigned  n_bits=32  bin_pt=0  quantization="Round  (unbiased: +/- Inf)"  overflow=Saturate  period=4  dbl_ovrd=off  timing_constraint=None  locs_specified=off  LOCs={}  block_type=gatewayin
      settings: infoedit="Gateway in block.  Converts inputs of type Simulink integer, double and fixed point to  Xilinx fixed point type.<P><P>Hardware notes:  In hardware these blocks become top level input ports."  block_version=VER_STRING_GOES_HERE
  SMWOWE_RxModulation : xbsIndex_r4/Gateway In  arith_type=Boolean  n_bits=1  bin_pt=0  quantization="Round  (unbiased: +/- Inf)"  overflow=Saturate  period=4  dbl_ovrd=off  timing_constraint=None  locs_specified=off  LOCs={}  block_type=gatewayin
      settings: infoedit="Gateway in block.  Converts inputs of type Simulink integer, double and fixed point to  Xilinx fixed point type.<P><P>Hardware notes:  In hardware these blocks become top level input ports."  block_version=VER_STRING_GOES_HERE
  SMWO_RxModulation : xbsIndex_r4/Dual Port RAM  depth=numSubcarriers*2  initVector=subcarrier_QAM_Values  distributed_mem=Block RAM  init_a=0  init_b=0  rst_a=off  rst_b=off  en_a=off  en_b=off  latency=1  write_mode_A=Read After Write  write_mode_B=Read After Write  dbl_ovrd=off  optimize=Area  use_rpm=on  block_type=dpram
      settings: block_version=VER_STRING_GOES_HERE
  Terminator : Terminator
  mod : Outport
  wires:
  Xk_index/1 -> Addr Gen/1(Xk_index)
  Sym Index/1 -> Addr Gen/2(Sym Index)
  Addr Gen/2(IgnoreSym) -> Inverter/1
  Concat/1 -> Logical/2
  SMWO_RxModulation/2 -> Terminator/1
  SMWODataI_RxModulation/1 -> SMWO_RxModulation/5
  SMWOWE_RxModulation/1 -> SMWO_RxModulation/6
  SMWOAddr_RxModulation/1 -> SMWO_RxModulation/4
  Constant/1 -> SMWOAddr_RxModulation/1, SMWODataI_RxModulation/1, SMWOWE_RxModulation/1
  Constant7/1 -> SMWO_RxModulation/2
  Constant4/1 -> SMWO_RxModulation/3
  Inverter/1 -> Concat/4, Concat/3, Concat/2, Concat/1
  Addr Gen/3(Mod Addr) -> SMWO_RxModulation/1
  SMWO_RxModulation/1 -> Logical/3
  Logical/1 -> mod/1
  [regRx_simpleDynRxModulationEn] -> Inverter1/1
  Logical1/1 -> Concat1/1, Concat1/2, Concat1/3, Concat1/4
  FullRateMask/1 -> 4LSB/1
  Logical3/1 -> Logical/1
  4LSB/1 -> Logical3/2
  Concat1/1 -> Logical3/1
  Inverter1/1 -> Logical1/1
  Addr Gen/1(BaseRate) -> Logical1/2

== system ofdm_txrx_alamouti/OFDM Rx Alamouti/Equalizer & Packetizer/Packet_Constructor/Slicer ==
  note: 8MSB
  note: 8MSB-7
  SliceSel : Inport
  D : Inport  Port=2
  " byte mux1" : xbsIndex_r4/Mux  inputs=8  en=off  latency=0  precision=Full  arith_type="Signed  (2's comp)"  n_bits=8  bin_pt=2  quantization=Truncate  overflow=Wrap  dbl_ovrd=off  block_type=mux
      settings: xl_area=[16 0 0 32 0 0 0]  block_version=VER_STRING_GOES_HERE
  Slice : xbsIndex_r4/Slice  nbits=8  boolean_output=off  mode=Lower Bit Location + Width  bit1=0  base1=MSB of Input  bit0=0  base0=LSB of Input  dbl_ovrd=off  block_type=slice
      settings: infoedit="Extracts a given range of bits from each input sample and presents it at the output.  The output type is ordinarily unsigned with binary point at zero, but can be Boolean when the slice is one bit wide.<P><P>Hardware notes: In hardware this block costs nothing."  block_version=VER_STRING_GOES_HERE
  Slice1 : xbsIndex_r4/Slice  nbits=8  boolean_output=off  mode=Lower Bit Location + Width  bit1=0  base1=MSB of Input  bit0=1  base0=LSB of Input  dbl_ovrd=off  block_type=slice
      settings: infoedit="Extracts a given range of bits from each input sample and presents it at the output.  The output type is ordinarily unsigned with binary point at zero, but can be Boolean when the slice is one bit wide.<P><P>Hardware notes: In hardware this block costs nothing."  block_version=VER_STRING_GOES_HERE
  Slice2 : xbsIndex_r4/Slice  nbits=8  boolean_output=off  mode=Lower Bit Location + Width  bit1=0  base1=MSB of Input  bit0=2  base0=LSB of Input  dbl_ovrd=off  block_type=slice
      settings: infoedit="Extracts a given range of bits from each input sample and presents it at the output.  The output type is ordinarily unsigned with binary point at zero, but can be Boolean when the slice is one bit wide.<P><P>Hardware notes: In hardware this block costs nothing."  block_version=VER_STRING_GOES_HERE
  Slice3 : xbsIndex_r4/Slice  nbits=8  boolean_output=off  mode=Lower Bit Location + Width  bit1=0  base1=MSB of Input  bit0=3  base0=LSB of Input  dbl_ovrd=off  block_type=slice
      settings: infoedit="Extracts a given range of bits from each input sample and presents it at the output.  The output type is ordinarily unsigned with binary point at zero, but can be Boolean when the slice is one bit wide.<P><P>Hardware notes: In hardware this block costs nothing."  block_version=VER_STRING_GOES_HERE
  Slice4 : xbsIndex_r4/Slice  nbits=8  boolean_output=off  mode=Lower Bit Location + Width  bit1=0  base1=MSB of Input  bit0=4  base0=LSB of Input  dbl_ovrd=off  block_type=slice
      settings: infoedit="Extracts a given range of bits from each input sample and presents it at the output.  The output type is ordinarily unsigned with binary point at zero, but can be Boolean when the slice is one bit wide.<P><P>Hardware notes: In hardware this block costs nothing."  block_version=VER_STRING_GOES_HERE
  Slice5 : xbsIndex_r4/Slice  nbits=8  boolean_output=off  mode=Lower Bit Location + Width  bit1=0  base1=MSB of Input  bit0=5  base0=LSB of Input  dbl_ovrd=off  block_type=slice
      settings: infoedit="Extracts a given range of bits from each input sample and presents it at the output.  The output type is ordinarily unsigned with binary point at zero, but can be Boolean when the slice is one bit wide.<P><P>Hardware notes: In hardware this block costs nothing."  block_version=VER_STRING_GOES_HERE
  Slice6 : xbsIndex_r4/Slice  nbits=8  boolean_output=off  mode=Lower Bit Location + Width  bit1=0  base1=MSB of Input  bit0=6  base0=LSB of Input  dbl_ovrd=off  block_type=slice
      settings: infoedit="Extracts a given range of bits from each input sample and presents it at the output.  The output type is ordinarily unsigned with binary point at zero, but can be Boolean when the slice is one bit wide.<P><P>Hardware notes: In hardware this block costs nothing."  block_version=VER_STRING_GOES_HERE
  Slice7 : xbsIndex_r4/Slice  nbits=8  boolean_output=off  mode=Lower Bit Location + Width  bit1=0  base1=MSB of Input  bit0=7  base0=LSB of Input  dbl_ovrd=off  block_type=slice
      settings: infoedit="Extracts a given range of bits from each input sample and presents it at the output.  The output type is ordinarily unsigned with binary point at zero, but can be Boolean when the slice is one bit wide.<P><P>Hardware notes: In hardware this block costs nothing."  block_version=VER_STRING_GOES_HERE
  Q : Outport
  wires:
  " byte mux1"/1 -> Q/1
  SliceSel/1 -> " byte mux1"/1
  D/1 -> Slice3/1, Slice6/1, Slice7/1, Slice5/1, Slice4/1, Slice2/1, Slice1/1, Slice/1
  Slice/1 -> " byte mux1"/2
  Slice1/1 -> " byte mux1"/3
  Slice2/1 -> " byte mux1"/4
  Slice3/1 -> " byte mux1"/5
  Slice4/1 -> " byte mux1"/6
  Slice5/1 -> " byte mux1"/7
  Slice6/1 -> " byte mux1"/8
  Slice7/1 -> " byte mux1"/9

== system ofdm_txrx_alamouti/OFDM Rx Alamouti/Equalizer & Packetizer/Phase Adjust/Bus Creator ==
  AA-I : Inport
  AA-Q : Inport  Port=2
  BA-I : Inport  Port=3
  BA-Q : Inport  Port=4
  Bus Creator1 : BusCreator  Inputs=2
  Bus Creator2 : BusCreator  Inputs=2
  I : Outport
  Q : Outport  Port=2
  wires:
  Bus Creator1/1 -> I/1
  Bus Creator2/1 -> Q/1
  AA-Q/1 -> Bus Creator2/1
  BA-Q/1 -> Bus Creator2/2
  AA-I/1 -> Bus Creator1/1
  BA-I/1 -> Bus Creator1/2

== system ofdm_txrx_alamouti/OFDM Rx Alamouti/Equalizer & Packetizer/Phase Adjust/Bus Creator1 ==
  R0_I : Inport
  R0_Q : Inport  Port=2
  R1_I : Inport  Port=3
  R1_Q : Inport  Port=4
  Bus Creator1 : BusCreator  Inputs=2
  Bus Creator2 : BusCreator  Inputs=2
  " I" : Outport
  " Q" : Outport  Port=2
  wires:
  Bus Creator1/1 -> " I"/1
  Bus Creator2/1 -> " Q"/1
  R0_I/1 -> Bus Creator1/1
  R1_I/1 -> Bus Creator1/2
  R0_Q/1 -> Bus Creator2/1
  R1_Q/1 -> Bus Creator2/2

== system ofdm_txrx_alamouti/OFDM Rx Alamouti/Equalizer & Packetizer/Phase Adjust/Bus Selector ==
  H-I : Inport
  H-Q : Inport  Port=2
  Bus Selector1 : BusSelector  OutputSignals=signal1,signal2
  Bus Selector2 : BusSelector  OutputSignals=signal1,signal2
  AA_I : Outport
  AA_Q : Outport  Port=2
  BA_I : Outport  Port=3
  BA_Q : Outport  Port=4
  wires:
  H-I/1 -> Bus Selector2/1
  H-Q/1 -> Bus Selector1/1
  Bus Selector1/1 -> AA_Q/1  "<signal1>"
  Bus Selector1/2 -> BA_Q/1  "<signal2>"
  Bus Selector2/1 -> AA_I/1  "<signal1>"
  Bus Selector2/2 -> BA_I/1  "<signal2>"

== system ofdm_txrx_alamouti/OFDM Rx Alamouti/Equalizer & Packetizer/Phase Adjust/Bus Selector2 ==
  Y_I : Inport
  Y_Q : Inport  Port=2
  Bus Selector1 : BusSelector  OutputSignals=signal1,signal2
  Bus Selector2 : BusSelector  OutputSignals=signal1,signal2
  R0_I : Outport
  R0_Q : Outport  Port=2
  R1_I : Outport  Port=3
  R1_Q : Outport  Port=4
  wires:
  Bus Selector1/1 -> R0_I/1  "<signal1>"
  Y_I/1 -> Bus Selector1/1
  Bus Selector1/2 -> R1_I/1  "<signal2>"
  Bus Selector2/1 -> R0_Q/1  "<signal1>"
  Y_Q/1 -> Bus Selector2/1
  Bus Selector2/2 -> R1_Q/1  "<signal2>"
